simulink model "r_2x_8a_16x_4k_r352"  (Simulink 8.0) (continued)
== system r_2x_8a_16x_4k_r352 == (continued)
  Slice2 : xbsIndex_r4/Slice  nbits=1  boolean_output=on  mode=Lower Bit Location + Width  bit1=0  base1=MSB of Input  bit0=10  base0=LSB of Input  dbl_ovrd=off  block_type=slice
      settings: infoedit="Extracts a given range of bits from each input sample and presents it at the output.  The output type is ordinarily unsigned with binary point at zero, but can be Boolean when the slice is one bit wide.<P><P>Hardware notes: In hardware this block costs nothing."  block_version=10.1.3
  Slice3 : xbsIndex_r4/Slice  nbits=2  boolean_output=off  mode=Lower Bit Location + Width  bit1=0  base1=MSB of Input  bit0=1  base0=LSB of Input  dbl_ovrd=off  block_type=slice
      settings: infoedit="Extracts a given range of bits from each input sample and presents it at the output.  The output type is ordinarily unsigned with binary point at zero, but can be Boolean when the slice is one bit wide.<P><P>Hardware notes: In hardware this block costs nothing."  block_version=10.1.3
  Slice4 : xbsIndex_r4/Slice  nbits=1  boolean_output=on  mode=Upper Bit Location + Width  bit1=0  base1=MSB of Input  bit0=0  base0=LSB of Input  dbl_ovrd=off  block_type=slice
      settings: block_version=10.1.3
  Slice5 : xbsIndex_r4/Slice  nbits=6  boolean_output=off  mode=Lower Bit Location + Width  bit1=0  base1=MSB of Input  bit0=9  base0=LSB of Input  dbl_ovrd=off  block_type=slice
      settings: infoedit="Extracts a given range of bits from each input sample and presents it at the output.  The output type is ordinarily unsigned with binary point at zero, but can be Boolean when the slice is one bit wide.<P><P>Hardware notes: In hardware this block costs nothing."  block_version=10.1.3
  Slice6 : xbsIndex_r4/Slice  nbits=1  boolean_output=on  mode=Lower Bit Location + Width  bit1=0  base1=MSB of Input  bit0=12  base0=LSB of Input  dbl_ovrd=off  block_type=slice
      settings: block_version=10.1.3
  Slice7 : xbsIndex_r4/Slice  nbits=1  boolean_output=on  mode=Lower Bit Location + Width  bit1=0  base1=MSB of Input  bit0=8  base0=LSB of Input  dbl_ovrd=off  block_type=slice
      settings: infoedit="Extracts a given range of bits from each input sample and presents it at the output.  The output type is ordinarily unsigned with binary point at zero, but can be Boolean when the slice is one bit wide.<P><P>Hardware notes: In hardware this block costs nothing."  block_version=10.1.3
  Slice8 : xbsIndex_r4/Slice  nbits=1  boolean_output=on  mode=Lower Bit Location + Width  bit1=0  base1=MSB of Input  bit0=11  base0=LSB of Input  dbl_ovrd=off  block_type=slice
      settings: infoedit="Extracts a given range of bits from each input sample and presents it at the output.  The output type is ordinarily unsigned with binary point at zero, but can be Boolean when the slice is one bit wide.<P><P>Hardware notes: In hardware this block costs nothing."  block_version=10.1.3
  Slice9 : xbsIndex_r4/Slice  nbits=1  boolean_output=on  mode=Lower Bit Location + Width  bit1=0  base1=MSB of Input  bit0=15  base0=LSB of Input  dbl_ovrd=off  block_type=slice
      settings: infoedit="Extracts a given range of bits from each input sample and presents it at the output.  The output type is ordinarily unsigned with binary point at zero, but can be Boolean when the slice is one bit wide.<P><P>Hardware notes: In hardware this block costs nothing."  block_version=10.1.3
  Subsystem : Subsystem  mask{payload_len=32}
      mask prompts: payload_len "Payload length?"
      mask init:
      | packet_len = payload_len + 1;
      | packet_bits = ceil(log2(packet_len));
      | payload_bits = ceil(log2(payload_len));
  Subsystem1 : Subsystem
  Subsystem14 : Subsystem
  Subsystem2 : Subsystem
  Subsystem3 : Subsystem
  Subsystem4 : Subsystem
  Subsystem5 : Subsystem
  Subsystem6 : Subsystem
  Subsystem7 : Subsystem
  Terminator{1..27,30} (28 alike) : Terminator
  XSG core config : xps_library/XSG core config  Tag=xps:xsg  hw_sys=ROACH:sx95t  clk_src=arb_clk  ROACH_clk_src=arb_clk  ROACH2_clk_src=adc0_clk  clk_rate=210  sample_period=1  synthesis_tool=XST
  acc_len : xps_library/software register  Tag=xps:sw_reg  io_dir=From Processor  arith_type=Unsigned  bitwidth=32  bin_pt=0  sample_period=1  gw_name=r_2x_8a_16x_4k_r352_acc_len_user_data_out
  board_id : xps_library/software register  Tag=xps:sw_reg  io_dir=From Processor  arith_type=Unsigned  bitwidth=32  bin_pt=0  sample_period=1  gw_name=r_2x_8a_16x_4k_r352_board_id_user_data_out
  ctrl : xps_library/software register  Tag=xps:sw_reg  io_dir=From Processor  arith_type=Unsigned  bitwidth=32  bin_pt=0  sample_period=1  gw_name=r_2x_8a_16x_4k_r352_ctrl_user_data_out
  delay1 : xbsIndex_r4/Delay  rst=off  infoeditControl=Selection of Reset will increase slice count due to use of real FFs and instead of SRLs  en=off  latency=2  dbl_ovrd=off  reg_retiming=on  block_type=delay
  delay{2,4,6..17,19..20} (16 alike) : xbsIndex_r4/Delay  rst=off  infoeditControl=Selection of Reset will increase slice count due to use of real FFs and instead of SRLs  en=off  latency=1  dbl_ovrd=off  reg_retiming=on  block_type=delay
  delay5 : xbsIndex_r4/Delay  rst=off  infoeditControl=Selection of Reset will increase slice count due to use of real FFs and instead of SRLs  en=off  latency=2  dbl_ovrd=off  reg_retiming=on  block_type=delay
  dev_sel2 : xbsIndex_r4/Slice  nbits=1  boolean_output=off  mode=Lower Bit Location + Width  bit1=0  base1=MSB of Input  bit0=0  base0=LSB of Input  dbl_ovrd=off  block_type=slice
      settings: infoedit="Extracts a given range of bits from each input sample and presents it at the output.  The output type is ordinarily unsigned with binary point at zero, but can be Boolean when the slice is one bit wide.<P><P>Hardware notes: In hardware this block costs nothing."  block_version=10.1.3
  dev_sel3 : xbsIndex_r4/Slice  nbits=1  boolean_output=on  mode=Lower Bit Location + Width  bit1=0  base1=MSB of Input  bit0=0  base0=LSB of Input  dbl_ovrd=off  block_type=slice
      settings: infoedit="Extracts a given range of bits from each input sample and presents it at the output.  The output type is ordinarily unsigned with binary point at zero, but can be Boolean when the slice is one bit wide.<P><P>Hardware notes: In hardware this block costs nothing."  block_version=10.1.3
  dev_sel5 : xbsIndex_r4/Slice  nbits=2  boolean_output=off  mode=Lower Bit Location + Width  bit1=0  base1=MSB of Input  bit0=3  base0=LSB of Input  dbl_ovrd=off  block_type=slice
      settings: infoedit="Extracts a given range of bits from each input sample and presents it at the output.  The output type is ordinarily unsigned with binary point at zero, but can be Boolean when the slice is one bit wide.<P><P>Hardware notes: In hardware this block costs nothing."  block_version=10.1.3
  err_clear_sim{1..3,5..9} (8 alike) : Constant  Value=0
  front_panel : Subsystem  mask{in_step_period=16, desired_step_period=23}
      mask prompts: in_step_period "Input step period (2^?)"; desired_step_period "Desired step period (2^?)"
  gbe0 : xps_library/ten_Gbe_v2  Tag=xps:tengbe_v2  flavour=cx4  slot=1  port_r1=0  port_r2_cx4=0  port_r2_sfpp=0  rx_dist_ram=on  large_frames=off  show_param=on  pre_emph_r2=0.74  pre_emph=3  post_emph_r2=0.18  rxeqmix_r2=7  swing_r2=940  swing=800  fab_en=off  fab_mac=hex2dec('123456780000')  fab_ip=192*(2^24) + 168*(2^16) + 5*(2^8) + 20*(2^0)  fab_udp=10000  fab_gate=1  cpu_rx_en=on  cpu_tx_en=on
  gbe_err : Subsystem  mask{payload_len=32}
      mask prompts: payload_len "Packet payload length in 64-bit words?"
      mask init:
      | packet_len = payload_len + 1;
      | packet_bits = ceil(log2(packet_len));
      | payload_bits = ceil(log2(payload_len));
  gbe_out_ip : xps_library/software register  Tag=xps:sw_reg  io_dir=From Processor  arith_type=Unsigned  bitwidth=32  bin_pt=0  sample_period=1  gw_name=r_2x_8a_16x_4k_r352_gbe_out_ip_user_data_out
  gbe_out_port : xps_library/software register  Tag=xps:sw_reg  io_dir=From Processor  arith_type=Unsigned  bitwidth=32  bin_pt=0  sample_period=1  gw_name=r_2x_8a_16x_4k_r352_gbe_out_port_user_data_out
  gbe_rx_cnt0 : xps_library/software register  Tag=xps:sw_reg  io_dir=To Processor  arith_type=Unsigned  bitwidth=32  bin_pt=0  sample_period=1  gw_name=r_2x_8a_16x_4k_r352_gbe_rx_cnt0_user_data_in
  gbe_rx_err_cnt0 : xps_library/software register  Tag=xps:sw_reg  io_dir=To Processor  arith_type=Unsigned  bitwidth=32  bin_pt=0  sample_period=1  gw_name=r_2x_8a_16x_4k_r352_gbe_rx_err_cnt0_user_data_in
  gbe_tx_cnt0 : xps_library/software register  Tag=xps:sw_reg  io_dir=To Processor  arith_type=Unsigned  bitwidth=32  bin_pt=0  sample_period=1  gw_name=r_2x_8a_16x_4k_r352_gbe_tx_cnt0_user_data_in
  gbe_tx_err_cnt0 : xps_library/software register  Tag=xps:sw_reg  io_dir=To Processor  arith_type=Unsigned  bitwidth=32  bin_pt=0  sample_period=1  gw_name=r_2x_8a_16x_4k_r352_gbe_tx_err_cnt0_user_data_in
  last_missing_ant0 : xps_library/software register  Tag=xps:sw_reg  io_dir=To Processor  arith_type=Unsigned  bitwidth=32  bin_pt=0  sample_period=1  gw_name=r_2x_8a_16x_4k_r352_last_missing_ant0_user_data_in
  last_missing_ant1 : xps_library/software register  Tag=xps:sw_reg  io_dir=To Processor  arith_type=Unsigned  bitwidth=32  bin_pt=0  sample_period=1  gw_name=r_2x_8a_16x_4k_r352_last_missing_ant1_user_data_in
  led0_10gbe_fault : xps_library/gpio  Tag=xps:gpio  io_group=ROACH:led  io_dir=out  arith_type=Boolean  bitwidth=1  bin_pt=0  bit_index=0  sample_period=1  use_single_ended=off  use_ddr=off  reg_iob=off  reg_clk_phase=0  termination=None
      link override convert: arith_type=Boolean  n_bits=1  bin_pt=0
  led1_xaui_sync : xps_library/gpio  Tag=xps:gpio  io_group=ROACH:led  io_dir=out  arith_type=Boolean  bitwidth=1  bin_pt=0  bit_index=1  sample_period=1  use_single_ended=off  use_ddr=off  reg_iob=off  reg_clk_phase=0  termination=None
      link override convert: arith_type=Boolean  n_bits=1  bin_pt=0
  led2_x_sync : xps_library/gpio  Tag=xps:gpio  io_group=ROACH:led  io_dir=out  arith_type=Boolean  bitwidth=1  bin_pt=0  bit_index=2  sample_period=1  use_single_ended=off  use_ddr=off  reg_iob=off  reg_clk_phase=0  termination=None
      link override convert: arith_type=Boolean  n_bits=1  bin_pt=0
  led3_new_acc : xps_library/gpio  Tag=xps:gpio  io_group=ROACH:led  io_dir=out  arith_type=Boolean  bitwidth=1  bin_pt=0  bit_index=3  sample_period=1  use_single_ended=off  use_ddr=off  reg_iob=off  reg_clk_phase=0  termination=None
      link override convert: arith_type=Boolean  n_bits=1  bin_pt=0
  master_reset_sim4 : Constant  Value=2
  miss_cnt0 : Subsystem  mask{n_ants_bits=3, xeng_int_bits=7}
      mask prompts: n_ants_bits "Number of antennas in system (2^?)"; xeng_int_bits "X engine integration time (2^?)"
  miss_cnt1 : Subsystem  mask{n_ants_bits=3, xeng_int_bits=7}
      mask prompts: n_ants_bits "Number of antennas in system (2^?)"; xeng_int_bits "X engine integration time (2^?)"
  mux_out : Subsystem  mask{idle_clks=62000}
      mask prompts: idle_clks "Rate control. Idle clocks between packets"
      mask init:
      | idle_clks_bits = ceil(log2(idle_clks));
  negedge_delay{,1..4} (5 alike) : casper_library_misc/negedge_delay  pulse_len=2^23
  pack_out0 : Subsystem  mask(pkt_pack_mask){vector_len=147456/2, heap_len=147456/2*16*4, fifo_latency=2, spead_msw=64, spead_lsw=40, spead_item=6144, pkt_bits=9}
      mask prompts: vector_len "vector length"; heap_len "System heap size"; fifo_latency "Fifo Latency"; spead_msw "SPEAD flavour (MSw)"; spead_lsw "SPEAD flavour (LSw)"; spead_item "SPEAD item number"; pkt_bits "desired payload length (2^?) 64-bit words"
      mask help:
      | This block generates packets from a datastream.
      |
      | Packets are created with a fixed header followed by a user-specified number of 64-bit options.
      |
      | Requires a 64-bit data stream.
      |
      | See the documentation for further details.
      mask init:
      | vector_bits = ceil(log2(vector_len));
      | pkt_len=2^(pkt_bits);
  pack_out1 : Subsystem  mask(pkt_pack_mask){vector_len=147456/2, heap_len=147456/2*16*4, fifo_latency=2, spead_msw=64, spead_lsw=40, spead_item=6144, pkt_bits=9}
      mask prompts: vector_len "vector length"; heap_len "System heap size"; fifo_latency "Fifo Latency"; spead_msw "SPEAD flavour (MSw)"; spead_lsw "SPEAD flavour (LSw)"; spead_item "SPEAD item number"; pkt_bits "desired payload length (2^?) 64-bit words"
      mask help:
      | This block generates packets from a datastream.
      |
      | Packets are created with a fixed header followed by a user-specified number of 64-bit options.
      |
      | Requires a 64-bit data stream.
      |
      | See the documentation for further details.
      mask init:
      | vector_bits = ceil(log2(vector_len));
      | pkt_len=2^(pkt_bits);
  pack_out_cnt0 : xps_library/software register  Tag=xps:sw_reg  io_dir=To Processor  arith_type=Unsigned  bitwidth=32  bin_pt=0  sample_period=1  gw_name=r_2x_8a_16x_4k_r352_pack_out_cnt0_user_data_in
  pack_out_cnt1 : xps_library/software register  Tag=xps:sw_reg  io_dir=To Processor  arith_type=Unsigned  bitwidth=32  bin_pt=0  sample_period=1  gw_name=r_2x_8a_16x_4k_r352_pack_out_cnt1_user_data_in
  pack_out_err0 : xps_library/software register  Tag=xps:sw_reg  io_dir=To Processor  arith_type=Unsigned  bitwidth=32  bin_pt=0  sample_period=1  gw_name=r_2x_8a_16x_4k_r352_pack_out_err0_user_data_in
  pack_out_err1 : xps_library/software register  Tag=xps:sw_reg  io_dir=To Processor  arith_type=Unsigned  bitwidth=32  bin_pt=0  sample_period=1  gw_name=r_2x_8a_16x_4k_r352_pack_out_err1_user_data_in
  pkt_reord0 : Subsystem  mask{ant_bits=3, xeng_bits=4, chan_bits=12, x_int_bits=7, window_bits=3, min_sync_bits=10, share_bits=1, nx=0}
      mask prompts: ant_bits "Maximum Number of Antennas (2^?):"; xeng_bits "Number of Xengines in the system (2^?)"; chan_bits "Number of frequency channels in the system (2^?)"; x_int_bits "X Engine Integration Length (2^?):"; window_bits "Number of Windows to Buffer (2^?):"; min_sync_bits "Minimum sync period (2^n)"; share_bits "Number of Xengines on this link (2^?)"; nx "This is engine number"
      mask init:
      | sync_bits=max([min_sync_bits,chan_bits+x_int_bits+ant_bits-xeng_bits]);
      | %sync_bits=chan_bits+x_int_bits+ant_bits-xeng_bits;
      | fmtstr = sprintf('Xeng %d\nSync period: 2^%d\nPayload Len: %i', nx,sync_bits,2^(x_int_bits - 2));
      | set_param(gcb, 'AttributesFormatString', fmtstr);
      settings: AttributesFormatString=|
      | Xeng 0
      | Sync period: 2^18
      | Payload Len: 32
  pkt_reord1 : Subsystem  mask{ant_bits=3, xeng_bits=4, chan_bits=12, x_int_bits=7, window_bits=3, min_sync_bits=10, share_bits=1, nx=1}
      mask prompts: ant_bits "Maximum Number of Antennas (2^?):"; xeng_bits "Number of Xengines in the system (2^?)"; chan_bits "Number of frequency channels in the system (2^?)"; x_int_bits "X Engine Integration Length (2^?):"; window_bits "Number of Windows to Buffer (2^?):"; min_sync_bits "Minimum sync period (2^n)"; share_bits "Number of Xengines on this link (2^?)"; nx "This is engine number"
      mask init: same as r_2x_8a_16x_4k_r352/pkt_reord0
      settings: AttributesFormatString=|
      | Xeng 1
      | Sync period: 2^18
      | Payload Len: 32
  pkt_reord_cnt0 : xps_library/software register  Tag=xps:sw_reg  io_dir=To Processor  arith_type=Unsigned  bitwidth=32  bin_pt=0  sample_period=1  gw_name=r_2x_8a_16x_4k_r352_pkt_reord_cnt0_user_data_in
  pkt_reord_cnt1 : xps_library/software register  Tag=xps:sw_reg  io_dir=To Processor  arith_type=Unsigned  bitwidth=32  bin_pt=0  sample_period=1  gw_name=r_2x_8a_16x_4k_r352_pkt_reord_cnt1_user_data_in
  pkt_reord_err0 : xps_library/software register  Tag=xps:sw_reg  io_dir=To Processor  arith_type=Unsigned  bitwidth=32  bin_pt=0  sample_period=1  gw_name=r_2x_8a_16x_4k_r352_pkt_reord_err0_user_data_in
  pkt_reord_err1 : xps_library/software register  Tag=xps:sw_reg  io_dir=To Processor  arith_type=Unsigned  bitwidth=32  bin_pt=0  sample_period=1  gw_name=r_2x_8a_16x_4k_r352_pkt_reord_err1_user_data_in
  posedge2 : casper_library_misc/posedge  Tag=Rising Edge Detector
  prep_snap_rx0 : Subsystem
  rcs : Subsystem  AncestorBlock=casper_library_misc/rcs  mask(rcs){app_src=timestamp, lib_src=git, user_val=352}
      mask prompts: app_src "Application revision info to capture"; lib_src "CASPER libraries revision info to capture"; user_val "User specified value"
      mask help:
      | Embeds revision control information for the user design and libraries.
      | On failure to locate specified revision information, falls back to
      | timestamps (current time for libraries).
      | A user specified value is also stored.
      mask init:
      | rcs_init(gcb, ...
      |     'app_src', app_src, ...
      |     'lib_src', lib_src);
      mask TypeOptions app_src: timestamp | git | svn
      mask TypeOptions lib_src: timestamp | git | svn
  rx_cnt0 : xps_library/software register  Tag=xps:sw_reg  io_dir=To Processor  arith_type=Unsigned  bitwidth=32  bin_pt=0  sample_period=1  gw_name=r_2x_8a_16x_4k_r352_rx_cnt0_user_data_in
  rx_err_cnt0 : xps_library/software register  Tag=xps:sw_reg  io_dir=To Processor  arith_type=Unsigned  bitwidth=32  bin_pt=0  sample_period=1  gw_name=r_2x_8a_16x_4k_r352_rx_err_cnt0_user_data_in
  snap_descramble0 : Subsystem  AncestorBlock=casper_library_scopes/snapshot  mask(snapshot){storage=bram, dram_dimm=1, dram_clock=200, nsamples=10, data_width=32, offset=on, circap=on, value=off, use_dsp48=on}
      mask prompts: storage "Storage medium"; dram_dimm "DRAM dimm"; dram_clock "DRAM clock rate"; nsamples "Number of Samples (2^?)"; data_width "Data width"; offset "Start delay support"; circap "Circular capture support"; value "Extra value capture support"; use_dsp48 "Use DSP48s to implement counters"
      mask help:
      | Standard snap block, but with the following optional extras;
      |  1) Optionally delay the fabric trigger by a user-specified number of valids.
      |  2) Capture continuously after a trigger until a stop pulse is received. Data is captured into BRAM continuously, with old data being overwritten until a stop command is received. Then stop capturing when memory is filled. This enables you to get some data before and some data after the stop pulse. Use the 'tr_en_cnt' register to determine where you are in your vector. 'Addr' register's MSb now indicates 'busy capturing'.
      |  3) Optionally capture a value on an input port to a register at the same time as the first data sample.
      |  4) Implement counters using DSP48Es to save resources.
      mask init:
      | snapshot_init(gcb, ...
      |     'storage', storage, ...
      |     'dram_dimm', dram_dimm, ...
      |     'dram_clock', dram_clock, ...
      |     'nsamples', nsamples, ...
      |     'data_width', data_width, ...
      |     'offset', offset, ...
      |     'circap', circap, ...
      |     'value', value, ...
      |     'use_dsp48', use_dsp48);
      mask TypeOptions storage: bram | dram
      mask Callback storage: snapshot_callback();
      mask TypeOptions dram_dimm: 1 | 2 | 3 | 4
      mask TypeOptions dram_clock: 200 | 250 | 266 | 300 | 333
      mask TypeOptions data_width: 8 | 16 | 32 | 64 | 128
  snap_descramble1 : Subsystem  AncestorBlock=casper_library_scopes/snapshot  mask(snapshot){storage=bram, dram_dimm=1, dram_clock=200, nsamples=10, data_width=32, offset=on, circap=on, value=off, use_dsp48=on}
      mask prompts: storage "Storage medium"; dram_dimm "DRAM dimm"; dram_clock "DRAM clock rate"; nsamples "Number of Samples (2^?)"; data_width "Data width"; offset "Start delay support"; circap "Circular capture support"; value "Extra value capture support"; use_dsp48 "Use DSP48s to implement counters"
      mask help:
      | Standard snap block, but with the following optional extras;
      |  1) Optionally delay the fabric trigger by a user-specified number of valids.
      |  2) Capture continuously after a trigger until a stop pulse is received. Data is captured into BRAM continuously, with old data being overwritten until a stop command is received. Then stop capturing when memory is filled. This enables you to get some data before and some data after the stop pulse. Use the 'tr_en_cnt' register to determine where you are in your vector. 'Addr' register's MSb now indicates 'busy capturing'.
      |  3) Optionally capture a value on an input port to a register at the same time as the first data sample.
      |  4) Implement counters using DSP48Es to save resources.
      mask init: same as r_2x_8a_16x_4k_r352/snap_descramble0
      mask TypeOptions storage: bram | dram
      mask Callback storage: snapshot_callback();
      mask TypeOptions dram_dimm: 1 | 2 | 3 | 4
      mask TypeOptions dram_clock: 200 | 250 | 266 | 300 | 333
      mask TypeOptions data_width: 8 | 16 | 32 | 64 | 128
  snap_gbe_rx0 : Subsystem  AncestorBlock=casper_library_scopes/snapshot  mask(snapshot){storage=bram, dram_dimm=1, dram_clock=200, nsamples=10, data_width=128, offset=off, circap=off, value=off, use_dsp48=on}
      mask prompts: storage "Storage medium"; dram_dimm "DRAM dimm"; dram_clock "DRAM clock rate"; nsamples "Number of Samples (2^?)"; data_width "Data width"; offset "Start delay support"; circap "Circular capture support"; value "Extra value capture support"; use_dsp48 "Use DSP48s to implement counters"
      mask help:
      | Standard snap block, but with the following optional extras;
      |  1) Optionally delay the fabric trigger by a user-specified number of valids.
      |  2) Capture continuously after a trigger until a stop pulse is received. Data is captured into BRAM continuously, with old data being overwritten until a stop command is received. Then stop capturing when memory is filled. This enables you to get some data before and some data after the stop pulse. Use the 'tr_en_cnt' register to determine where you are in your vector. 'Addr' register's MSb now indicates 'busy capturing'.
      |  3) Optionally capture a value on an input port to a register at the same time as the first data sample.
      |  4) Implement counters using DSP48Es to save resources.
      mask init: same as r_2x_8a_16x_4k_r352/snap_descramble0
      mask TypeOptions storage: bram | dram
      mask Callback storage: snapshot_callback();
      mask TypeOptions dram_dimm: 1 | 2 | 3 | 4
      mask TypeOptions dram_clock: 200 | 250 | 266 | 300 | 333
      mask TypeOptions data_width: 8 | 16 | 32 | 64 | 128
  snap_gbe_tx0 : Subsystem  AncestorBlock=casper_library_scopes/snapshot  mask(snapshot){storage=bram, dram_dimm=1, dram_clock=200, nsamples=10, data_width=128, offset=off, circap=off, value=off, use_dsp48=on}
      mask prompts: storage "Storage medium"; dram_dimm "DRAM dimm"; dram_clock "DRAM clock rate"; nsamples "Number of Samples (2^?)"; data_width "Data width"; offset "Start delay support"; circap "Circular capture support"; value "Extra value capture support"; use_dsp48 "Use DSP48s to implement counters"
      mask help:
      | Standard snap block, but with the following optional extras;
      |  1) Optionally delay the fabric trigger by a user-specified number of valids.
      |  2) Capture continuously after a trigger until a stop pulse is received. Data is captured into BRAM continuously, with old data being overwritten until a stop command is received. Then stop capturing when memory is filled. This enables you to get some data before and some data after the stop pulse. Use the 'tr_en_cnt' register to determine where you are in your vector. 'Addr' register's MSb now indicates 'busy capturing'.
      |  3) Optionally capture a value on an input port to a register at the same time as the first data sample.
      |  4) Implement counters using DSP48Es to save resources.
      mask init: same as r_2x_8a_16x_4k_r352/snap_descramble0
      mask TypeOptions storage: bram | dram
      mask Callback storage: snapshot_callback();
      mask TypeOptions dram_dimm: 1 | 2 | 3 | 4
      mask TypeOptions dram_clock: 200 | 250 | 266 | 300 | 333
      mask TypeOptions data_width: 8 | 16 | 32 | 64 | 128
  snap_rx0 : Subsystem  AncestorBlock=casper_library_scopes/snapshot  mask(snapshot){storage=bram, dram_dimm=1, dram_clock=200, nsamples=10, data_width=128, offset=off, circap=off, value=off, use_dsp48=on}
      mask prompts: storage "Storage medium"; dram_dimm "DRAM dimm"; dram_clock "DRAM clock rate"; nsamples "Number of Samples (2^?)"; data_width "Data width"; offset "Start delay support"; circap "Circular capture support"; value "Extra value capture support"; use_dsp48 "Use DSP48s to implement counters"
      mask help:
      | Standard snap block, but with the following optional extras;
      |  1) Optionally delay the fabric trigger by a user-specified number of valids.
      |  2) Capture continuously after a trigger until a stop pulse is received. Data is captured into BRAM continuously, with old data being overwritten until a stop command is received. Then stop capturing when memory is filled. This enables you to get some data before and some data after the stop pulse. Use the 'tr_en_cnt' register to determine where you are in your vector. 'Addr' register's MSb now indicates 'busy capturing'.
      |  3) Optionally capture a value on an input port to a register at the same time as the first data sample.
      |  4) Implement counters using DSP48Es to save resources.
      mask init: same as r_2x_8a_16x_4k_r352/snap_descramble0
      mask TypeOptions storage: bram | dram
      mask Callback storage: snapshot_callback();
      mask TypeOptions dram_dimm: 1 | 2 | 3 | 4
      mask TypeOptions dram_clock: 200 | 250 | 266 | 300 | 333
      mask TypeOptions data_width: 8 | 16 | 32 | 64 | 128
  snap_vacc0 : Subsystem  AncestorBlock=casper_library_scopes/snapshot  mask(snapshot){storage=bram, dram_dimm=1, dram_clock=200, nsamples=10, data_width=32, offset=on, circap=on, value=off, use_dsp48=on}
      mask prompts: storage "Storage medium"; dram_dimm "DRAM dimm"; dram_clock "DRAM clock rate"; nsamples "Number of Samples (2^?)"; data_width "Data width"; offset "Start delay support"; circap "Circular capture support"; value "Extra value capture support"; use_dsp48 "Use DSP48s to implement counters"
      mask help:
      | Standard snap block, but with the following optional extras;
      |  1) Optionally delay the fabric trigger by a user-specified number of valids.
      |  2) Capture continuously after a trigger until a stop pulse is received. Data is captured into BRAM continuously, with old data being overwritten until a stop command is received. Then stop capturing when memory is filled. This enables you to get some data before and some data after the stop pulse. Use the 'tr_en_cnt' register to determine where you are in your vector. 'Addr' register's MSb now indicates 'busy capturing'.
      |  3) Optionally capture a value on an input port to a register at the same time as the first data sample.
      |  4) Implement counters using DSP48Es to save resources.
      mask init: same as r_2x_8a_16x_4k_r352/snap_descramble0
      mask TypeOptions storage: bram | dram
      mask Callback storage: snapshot_callback();
      mask TypeOptions dram_dimm: 1 | 2 | 3 | 4
      mask TypeOptions dram_clock: 200 | 250 | 266 | 300 | 333
      mask TypeOptions data_width: 8 | 16 | 32 | 64 | 128
  snap_xeng0 : Subsystem  AncestorBlock=casper_library_scopes/snapshot  mask(snapshot){storage=bram, dram_dimm=1, dram_clock=200, nsamples=10, data_width=32, offset=on, circap=off, value=off, use_dsp48=on}
      mask prompts: storage "Storage medium"; dram_dimm "DRAM dimm"; dram_clock "DRAM clock rate"; nsamples "Number of Samples (2^?)"; data_width "Data width"; offset "Start delay support"; circap "Circular capture support"; value "Extra value capture support"; use_dsp48 "Use DSP48s to implement counters"
      mask help:
      | Standard snap block, but with the following optional extras;
      |  1) Optionally delay the fabric trigger by a user-specified number of valids.
      |  2) Capture continuously after a trigger until a stop pulse is received. Data is captured into BRAM continuously, with old data being overwritten until a stop command is received. Then stop capturing when memory is filled. This enables you to get some data before and some data after the stop pulse. Use the 'tr_en_cnt' register to determine where you are in your vector. 'Addr' register's MSb now indicates 'busy capturing'.
      |  3) Optionally capture a value on an input port to a register at the same time as the first data sample.
      |  4) Implement counters using DSP48Es to save resources.
      mask init: same as r_2x_8a_16x_4k_r352/snap_descramble0
      mask TypeOptions storage: bram | dram
      mask Callback storage: snapshot_callback();
      mask TypeOptions dram_dimm: 1 | 2 | 3 | 4
      mask TypeOptions dram_clock: 200 | 250 | 266 | 300 | 333
      mask TypeOptions data_width: 8 | 16 | 32 | 64 | 128
  tvg_sel : xps_library/software register  Tag=xps:sw_reg  io_dir=From Processor  arith_type=Unsigned  bitwidth=32  bin_pt=0  sample_period=1  gw_name=r_2x_8a_16x_4k_r352_tvg_sel_user_data_out
  unpack : Subsystem  mask{payload_len=32}
      mask prompts: payload_len "Expected payload length (in 64bit words)"
      mask init:
      | payload_bits = ceil(log2(payload_len)+1);
      | fmtstr = sprintf('Payload Len: %i', payload_len);
      | set_param(gcb, 'AttributesFormatString', fmtstr);
      settings: AttributesFormatString=Payload Len: 32
  vacc0 : Subsystem  mask{ants_bits=3, freq_bits=12, xeng_bits=4, which_qdr=qdr0}
      mask prompts: ants_bits "Number of antennas (2^n)"; freq_bits "Number of frequency channels (2^n)"; xeng_bits "Number of X engines (2^n)"; which_qdr "QDR"
      mask help: Hardcoded for X engines with a demux of 8!
      mask init:
      | vector_len = (2^ants_bits)*(2^ants_bits +1) /2 *8 * 2^(freq_bits-xeng_bits);
      | set_param([gcb, '/qv'], 'which_qdr', which_qdr);
      | fmtstr = sprintf('Vector Length: %d', vector_len);
      | set_param(gcb, 'AttributesFormatString', fmtstr);
      settings: AttributesFormatString=Vector Length: 73728
      mask TypeOptions which_qdr: qdr0 | qdr1
  vacc1 : Subsystem  mask{ants_bits=3, freq_bits=12, xeng_bits=4, which_qdr=qdr1}
      mask prompts: ants_bits "Number of antennas (2^n)"; freq_bits "Number of frequency channels (2^n)"; xeng_bits "Number of X engines (2^n)"; which_qdr "QDR"
      mask help: Hardcoded for X engines with a demux of 8!
      mask init: same as r_2x_8a_16x_4k_r352/vacc0
      settings: AttributesFormatString=Vector Length: 73728
      mask TypeOptions which_qdr: qdr0 | qdr1
  vacc_cnt0 : xps_library/software register  Tag=xps:sw_reg  io_dir=To Processor  arith_type=Unsigned  bitwidth=32  bin_pt=0  sample_period=1  gw_name=r_2x_8a_16x_4k_r352_vacc_cnt0_user_data_in
  vacc_cnt1 : xps_library/software register  Tag=xps:sw_reg  io_dir=To Processor  arith_type=Unsigned  bitwidth=32  bin_pt=0  sample_period=1  gw_name=r_2x_8a_16x_4k_r352_vacc_cnt1_user_data_in
  vacc_err_cnt0 : xps_library/software register  Tag=xps:sw_reg  io_dir=To Processor  arith_type=Unsigned  bitwidth=32  bin_pt=0  sample_period=1  gw_name=r_2x_8a_16x_4k_r352_vacc_err_cnt0_user_data_in
  vacc_err_cnt1 : xps_library/software register  Tag=xps:sw_reg  io_dir=To Processor  arith_type=Unsigned  bitwidth=32  bin_pt=0  sample_period=1  gw_name=r_2x_8a_16x_4k_r352_vacc_err_cnt1_user_data_in
  vacc_ld_status0 : xps_library/software register  Tag=xps:sw_reg  io_dir=To Processor  arith_type=Unsigned  bitwidth=32  bin_pt=0  sample_period=1  gw_name=r_2x_8a_16x_4k_r352_vacc_ld_status0_user_data_in
  vacc_ld_status1 : xps_library/software register  Tag=xps:sw_reg  io_dir=To Processor  arith_type=Unsigned  bitwidth=32  bin_pt=0  sample_period=1  gw_name=r_2x_8a_16x_4k_r352_vacc_ld_status1_user_data_in
  vacc_monitor : Subsystem
  vacc_monitor1 : Subsystem
  vacc_monitor2 : Subsystem
  vacc_monitor3 : Subsystem
  vacc_time_lsw : xps_library/software register  Tag=xps:sw_reg  io_dir=From Processor  arith_type=Unsigned  bitwidth=32  bin_pt=0  sample_period=1  gw_name=r_2x_8a_16x_4k_r352_vacc_time_lsw_user_data_out
  vacc_time_msw : xps_library/software register  Tag=xps:sw_reg  io_dir=From Processor  arith_type=Unsigned  bitwidth=32  bin_pt=0  sample_period=1  gw_name=r_2x_8a_16x_4k_r352_vacc_time_msw_user_data_out
  vacc_tvg0 : Subsystem  mask{len=147456/2}
      mask prompts: len "Number of Vectors"
      mask init:
      | fmtstr = sprintf('Vector Length: %d', len);
      | set_param(gcb, 'AttributesFormatString', fmtstr);
      settings: AttributesFormatString=Vector Length: 73728
  vacc_tvg1 : Subsystem  mask{len=147456/2}
      mask prompts: len "Number of Vectors"
      mask init:
      | fmtstr = sprintf('Vector Length: %d', len);
      | set_param(gcb, 'AttributesFormatString', fmtstr);
      settings: AttributesFormatString=Vector Length: 73728
  xeng0 : Subsystem  mask(xeng){n_ants=8, n_bits=4, acc_len=128, demux_factor=8, add_latency=1, mult_latency=2, bram_latency=2, use_ded_mult=1, use_bram_delay=1}
      mask prompts: n_ants "Number of antennas"; n_bits "Bit-width of samples in"; acc_len "Accumulation length"; add_latency "Adder latency"; mult_latency "Multiplier latency"; bram_latency "BRAM latency"; use_ded_mult "Implementation: Multiplier type (0=slices 1=embedded 2=bram)"; use_bram_delay "Implementation: Delay type (0=SLR, 1=BRAM)"
      mask help: CASPER X engine with added internal valid data masking functionality, descramble and integral demux. Based on Aaron Parsons' and Lynn Urry's original X engine design.
      mask init:
      | xeng_init(gcb, ...
      |     'n_ants', n_ants, ...
      |     'n_bits', n_bits, ...
      |     'acc_len', acc_len, ...
      |     'demux_factor', demux_factor, ...
      |     'add_latency', add_latency, ...
      |     'mult_latency', mult_latency, ...
      |     'bram_latency', bram_latency, ...
      |     'use_ded_mult', use_ded_mult, ...
      |     'use_bram_delay', use_bram_delay);
      settings: AttributesFormatString=n_ant=8, bits=4, mult=1, bram=1
      mask TypeOptions demux_factor: 1 | 2 | 4 | 8
  xeng1 : Subsystem  mask(xeng){n_ants=8, n_bits=4, acc_len=128, demux_factor=8, add_latency=1, mult_latency=2, bram_latency=2, use_ded_mult=1, use_bram_delay=1}
      mask prompts: n_ants "Number of antennas"; n_bits "Bit-width of samples in"; acc_len "Accumulation length"; add_latency "Adder latency"; mult_latency "Multiplier latency"; bram_latency "BRAM latency"; use_ded_mult "Implementation: Multiplier type (0=slices 1=embedded 2=bram)"; use_bram_delay "Implementation: Delay type (0=SLR, 1=BRAM)"
      mask help: CASPER X engine with added internal valid data masking functionality, descramble and integral demux. Based on Aaron Parsons' and Lynn Urry's original X engine design.
      mask init: same as r_2x_8a_16x_4k_r352/xeng0
      settings: AttributesFormatString=n_ant=8, bits=4, mult=1, bram=1
      mask TypeOptions demux_factor: 1 | 2 | 4 | 8
  xeng_tvg0 : Subsystem  AncestorBlock=casper_library/Correlator/xeng_tvg  mask{ant_bits=3, x_int_bits=7, sync_period=19}
      mask prompts: ant_bits "Number of Antennas (2^?):"; x_int_bits "X Integration Length (2^?):"; sync_period "Sync Pulse Period (2^?):"
  xeng_tvg1 : Subsystem  AncestorBlock=casper_library/Correlator/xeng_tvg  mask{ant_bits=3, x_int_bits=7, sync_period=19}
      mask prompts: ant_bits "Number of Antennas (2^?):"; x_int_bits "X Integration Length (2^?):"; sync_period "Sync Pulse Period (2^?):"
  xstatus0 : xps_library/software register  Tag=xps:sw_reg  io_dir=To Processor  arith_type=Unsigned  bitwidth=32  bin_pt=0  sample_period=1  gw_name=r_2x_8a_16x_4k_r352_xstatus0_user_data_in
  xstatus1 : xps_library/software register  Tag=xps:sw_reg  io_dir=To Processor  arith_type=Unsigned  bitwidth=32  bin_pt=0  sample_period=1  gw_name=r_2x_8a_16x_4k_r352_xstatus1_user_data_in
  wires:
  gbe_err/1(tx_cnt) -> Delay63/1(d)
  Subsystem/1(rx_cnt) -> Delay65/1(d)
  [cnt_rst] -> gbe_err/1(rst)
  [cnt_rst] -> Subsystem/1(rst)
  Subsystem5/1(rx_cnt) -> Delay66/1(d)
  [cnt_rst] -> Subsystem5/1(rst)
  unpack/2(valid) -> Delay37/1(d), Delay141/1(d), Delay80/1(d)
  unpack/4(mcnt) -> Delay39/1(d), Delay143/1(d)
  unpack/1(dout) -> Delay36/1(d), Delay140/1(d)
  unpack/3(eof) -> Delay38/1(d), Delay142/1(d), Delay81/1(d)
  unpack/5(ant) -> Delay35/1(d), Delay144/1(d)
  miss_cnt0/2(err_cnt) -> Delay62/1(d)
  [cnt_rst] -> miss_cnt0/1(rst)
  unpack/8(ip) -> Delay146/1(d)
  Delay36/1(q) -> pkt_reord0/1(din), pkt_reord1/1(din)
  Delay37/1(q) -> pkt_reord0/2(valid_in), pkt_reord1/2(valid_in)
  Delay38/1(q) -> pkt_reord0/3(eof), pkt_reord1/3(eof)
  Delay39/1(q) -> pkt_reord0/4(mcnt), pkt_reord1/4(mcnt)
  Delay35/1(q) -> pkt_reord0/5(ant), pkt_reord1/5(ant)
  Subsystem14/1(data) -> Delay41/1(d)
  Delay41/1(q) -> snap_descramble0/1(din)
  Subsystem14/2(we) -> Delay42/1(d)
  Subsystem14/3(trig) -> Delay43/1(d)
  Delay40/1(q) -> pkt_reord0/6(flag), pkt_reord1/6(flag)
  gbe_err/2(err_cnt) -> Delay64/1(d)
  Subsystem5/2(err_cnt) -> Delay67/1(d)
  miss_cnt0/1(spectr_cnt) -> Delay61/1(d)
  gbe_tx_cnt0/1 -> Terminator13/1
  gbe_tx_err_cnt0/1 -> Terminator14/1
  gbe_rx_cnt0/1 -> Terminator15/1
  rx_cnt0/1 -> Terminator16/1
  rx_err_cnt0/1 -> Terminator17/1
  pkt_reord_cnt0/1 -> Terminator25/1
  pkt_reord_err0/1 -> Terminator26/1
  Delay61/1(q) -> pkt_reord_cnt0/1
  Delay62/1(q) -> pkt_reord_err0/1
  Delay66/1(q) -> rx_cnt0/1
  Delay67/1(q) -> rx_err_cnt0/1
  Delay63/1(q) -> gbe_tx_cnt0/1
  Delay64/1(q) -> gbe_tx_err_cnt0/1
  Delay65/1(q) -> gbe_rx_cnt0/1
  pkt_reord0/4(mcnt_out) -> Delay26/1(d), Subsystem14/4(mcnt)
  pkt_reord0/1(sync_out) -> Delay20/1(d), [x_sync0], miss_cnt0/2(sync), Subsystem14/1(sync)
  pkt_reord0/3(valid_out) -> miss_cnt0/3(valid), Delay22/1(d), Subsystem14/3(valid)
  pkt_reord0/5(rcvd) -> miss_cnt0/4(rcvd), Subsystem14/6(rcvd)
  err_clear_sim3/1 -> board_id/1
  board_id/1 -> Delay72/1(d)
  Delay72/1(q) -> [board_id]
  master_reset_sim4/1 -> acc_len/1
  acc_len/1 -> [acc_len]
  tvg_sel/1 -> Delay89/1(d)
  Delay89/1(q) -> dev_sel2/1, Slice3/1, dev_sel5/1, Slice5/1
  dev_sel2/1 -> [xaui_tvg_sel]
  Slice3/1 -> [descr_tvg_sel]
  dev_sel5/1 -> [xeng_tvg_sel]
  Slice5/1 -> [vacc_tvg_sel]
  err_clear_sim2/1 -> Subsystem2/5(data_sel)
  err_clear_sim6/1 -> Subsystem2/4(valid_sel)
  err_clear_sim7/1 -> Subsystem2/3(inj_vector)
  err_clear_sim8/1 -> Subsystem2/2(inj_counter)
  Pulse Generator1/1 -> Subsystem2/1(new_acc)
  err_clear_sim9/1 -> Subsystem2/6(run)
  Subsystem2/1(Out1) -> tvg_sel/1
  Pulse Generator2/1 -> ctrl/1
  ctrl/1 -> Delay19/1(d)
  dev_sel3/1 -> Delay88/1(d)
  Slice9/1 -> Delay78/1(d)
  Slice7/1 -> posedge2/1
  posedge2/1 -> Delay58/1(d)
  Delay19/1(q) -> dev_sel3/1, Slice7/1, Slice1/1, Slice2/1, Slice8/1, Slice9/1, Slice14/1, Slice6/1, Slice10/1
  Delay58/1(q) -> [cnt_rst]
  Delay78/1(q) -> [gbe_rst]
  Delay88/1(q) -> [vacc_rst]
  Slice1/1 -> Delay60/1(d)
  Delay60/1(q) -> [gbe_enable]
  Delay17/1(q) -> negedge_delay/1
  [x_sync0] -> Delay17/1(d)
  led2_x_sync/1 -> Terminator30/1
  Constant3/1 -> Inverter2/1
  led1_xaui_sync/1 -> Terminator18/1
  led0_10gbe_fault/1 -> Terminator19/1
  Delay140/1(q) -> prep_snap_rx0/1(data_in)
  Delay141/1(q) -> prep_snap_rx0/2(valid_in)
  Delay142/1(q) -> prep_snap_rx0/3(eof_in)
  Delay143/1(q) -> prep_snap_rx0/4(mcnt)
  Delay144/1(q) -> prep_snap_rx0/5(ant)
  Delay145/1(q) -> prep_snap_rx0/6(flag)
  Delay146/1(q) -> prep_snap_rx0/7(ip_in)
  Delay147/1(q) -> prep_snap_rx0/8(loop_ack)
  Delay148/1(q) -> prep_snap_rx0/9(gbe_ack)
  Delay8/1(q) -> Subsystem4/7(bad_frame)
  Delay91/1(q) -> snap_descramble0/4(stop)
  Subsystem14/4(stop) -> Delay91/1(d)
  xeng_tvg0/1(sync_out) -> Delay56/1(d)
  xeng_tvg0/2(data_out) -> Delay73/1(d)
  xeng_tvg0/3(valid_out) -> Delay74/1(d)
  xeng0/1(sync_out) -> vacc_tvg0/2(sync_in), snap_xeng0/3(trig)
  xeng0/2(acc) -> 16b_32b/1(In1)
  xeng0/3(valid) -> snap_xeng0/2(we), vacc_tvg0/4(valid_in)
  [xeng_tvg_sel] -> xeng_tvg0/1(tvg_sel)
  [vacc_tvg_sel] -> vacc_tvg0/1(tvg_sel)
  Delay151/1(q) -> Delay83/1(d)
  Delay20/1(q) -> xeng_tvg0/2(sync)
  Delay21/1(q) -> xeng_tvg0/3(data_in)
  Delay22/1(q) -> xeng_tvg0/4(valid_in)
  Delay26/1(q) -> Delay151/1(d)
  [loopback_mux_rst] -> unpack/1(rst)
  gbe_rx_err_cnt0/1 -> Terminator7/1
  Delay2/1(q) -> gbe_rx_err_cnt0/1
  Subsystem/2(rx_err_cnt) -> Delay2/1(d)
  Slice2/1 -> Delay117/1(d)
  Delay117/1(q) -> [loopback_mux_rst]
  Delay118/1(q) -> negedge_delay1/1
  led3_new_acc/1 -> Terminator9/1
  Slice8/1 -> Delay122/1(d)
  Delay122/1(q) -> [gbe_out_rst]
  16b_32b/1(Out1) -> snap_xeng0/1(din), vacc_tvg0/3(data_in)
  miss_cnt0/3(last_missed_ant) -> Delay130/1(d)
  Slice14/1 -> Delay100/1(d)
  Delay100/1(q) -> [gbe_out_enable]
  unpack/6(flag) -> Delay40/1(d), Delay145/1(d)
  unpack/7(err) -> Delay82/1(d), [rx_bad_pkt]
  Delay12/1(q) -> Subsystem4/8(overrun)
  [gbe_up] -> Delay87/1(d)
  [gbe_rx] -> Delay86/1(d)
  [gbe_rxip] -> Delay75/1(d)
  [gbe_rxvld] -> Delay76/1(d)
  [gbe_rxdata] -> Delay79/1(d)
  [gbe_rxeof] -> Delay24/1(d)
  Delay87/1(q) -> Subsystem4/1(led_up)
  Delay86/1(q) -> Subsystem4/2(led_rx)
  Delay79/1(q) -> unpack/2(gbe_din)
  Delay76/1(q) -> unpack/3(gbe_valid)
  Delay75/1(q) -> unpack/4(gbe_ip)
  Delay24/1(q) -> unpack/5(gbe_eof)
  vacc_tvg0/1(sync_out) -> vacc0/5(sync_in)
  vacc_tvg0/2(data_out) -> vacc0/6(data_in)
  vacc_tvg0/3(valid_out) -> vacc0/7(we)
  [acc_len] -> vacc0/4(acc_len)
  xeng0/4(mcnt_out) -> delay5/1
  vacc_cnt0/1 -> Terminator10/1
  vacc_monitor/2(spec_cnt) -> vacc_cnt0/1
  vacc_err_cnt0/1 -> Terminator1/1
  vacc_monitor/1(error_cnt) -> vacc_err_cnt0/1
  vacc0/1(new_acc) -> [new_acc0]
  [cnt_rst] -> vacc_monitor/1(cnt_rst)
  vacc0/7(timestamp) -> delay7/1
  [cnt_rst] -> miss_cnt1/1(rst)
  xeng_tvg1/1(sync_out) -> Delay85/1(d)
  xeng_tvg1/2(data_out) -> Delay92/1(d)
  xeng_tvg1/3(valid_out) -> Delay94/1(d)
  xeng1/1(sync_out) -> vacc_tvg1/2(sync_in)
  xeng1/2(acc) -> 16b_32b1/1(In1)
  xeng1/3(valid) -> vacc_tvg1/4(valid_in)
  [xeng_tvg_sel] -> xeng_tvg1/1(tvg_sel)
  [vacc_tvg_sel] -> vacc_tvg1/1(tvg_sel)
  Delay30/1(q) -> Delay95/1(d)
  Delay49/1(q) -> xeng_tvg1/2(sync)
  Delay50/1(q) -> xeng_tvg1/3(data_in)
  Delay51/1(q) -> xeng_tvg1/4(valid_in)
  Delay52/1(q) -> Delay30/1(d)
  16b_32b1/1(Out1) -> vacc_tvg1/3(data_in)
  vacc_cnt1/1 -> Terminator20/1
  vacc_monitor1/2(spec_cnt) -> vacc_cnt1/1
  vacc_err_cnt1/1 -> Terminator8/1
  vacc_monitor1/1(error_cnt) -> vacc_err_cnt1/1
  [gbe_up] -> Subsystem3/5(link_up)
  [gbe_tx] -> Subsystem3/6(led_tx)
  [gbe_txd] -> Subsystem3/1(data_in)
  [gbe_txvld] -> Subsystem3/2(valid_in)
  [gbe_txip] -> Subsystem3/3(ip_in)
  [gbe_txeof] -> Subsystem3/4(eof_in)
  [gbe_rxvld] -> Delay25/1(d)
  [gbe_rxeof] -> Delay18/1(d)
  Delay25/1(q) -> Subsystem/2(valid)
  Delay18/1(q) -> Subsystem/3(eof)
  [gbe_rx_bad] -> Delay6/1(d)
  [gbe_rx_over] -> Delay7/1(d)
  [gbe_rxip] -> Delay53/1(d)
  [gbe_rxvld] -> Delay59/1(d)
  [gbe_rxdata] -> Delay70/1(d)
  [gbe_rxeof] -> Delay29/1(d)
  Delay70/1(q) -> Subsystem4/3(data_in)
  Delay59/1(q) -> Subsystem4/4(valid_in)
  Delay53/1(q) -> Subsystem4/5(ip_in)
  Delay29/1(q) -> Subsystem4/6(eof_in)
  [gbe_rxack] -> Subsystem4/9(ack_in)
  Delay80/1(q) -> Subsystem5/2(we)
  Delay81/1(q) -> Subsystem5/3(eof)
  Delay82/1(q) -> Subsystem5/4(flag)
  [gbe_tx_over] -> gbe_err/4(overflow)
  [gbe_txeof] -> gbe_err/3(eof)
  [gbe_txvld] -> gbe_err/2(valid)
  Delay6/1(q) -> Subsystem/4(rx_bad_frame)
  Delay7/1(q) -> Subsystem/5(rx_overflow)
  [gbe_rx_bad] -> Delay8/1(d)
  [gbe_rx_over] -> Delay12/1(d)
  [gbe_tx_full] -> Subsystem3/7(tx_full)
  [gbe_tx_over] -> Subsystem3/8(tx_over)
  Delay56/1(q) -> xeng0/1(sync_in)
  Delay73/1(q) -> xeng0/2(ant)
  Delay74/1(q) -> xeng0/3(window_valid)
  Delay83/1(q) -> xeng0/4(mcnt_in)
  Delay85/1(q) -> xeng1/1(sync_in)
  Delay92/1(q) -> xeng1/2(ant)
  Delay94/1(q) -> xeng1/3(window_valid)
  Delay95/1(q) -> xeng1/4(mcnt_in)
  [gbe_out_rst] -> delay8/1
  [gbe_txd] -> delay9/1
  [gbe_txvld] -> delay10/1
  [gbe_txip] -> delay11/1
  [gbe_out_txport] -> delay12/1
  [gbe_txeof] -> delay13/1
  [gbe_rxack] -> delay14/1
  [gbe_rxack] -> delay15/1
  gbe0/1 -> [gbe_up]
  gbe0/2 -> [gbe_rx]
  gbe0/3 -> [gbe_tx]
  gbe0/4 -> [gbe_tx_full]
  gbe0/5 -> [gbe_tx_over]
  gbe0/12 -> [gbe_rx_over]
  gbe0/11 -> [gbe_rx_bad]
  gbe0/6 -> [gbe_rxdata]
  gbe0/7 -> [gbe_rxvld]
  gbe0/8 -> [gbe_rxip]
  gbe0/9 -> [gbe_rxport]
  gbe0/10 -> [gbe_rxeof]
  Constant12/1 -> vacc_time_msw/1
  Constant6/1 -> vacc_time_lsw/1
  vacc_time_msw/1 -> Slice4/1, Slice13/1
  Slice13/1 -> Concat12/1
  vacc_time_lsw/1 -> Concat12/2
  Slice4/1 -> [vacc_ld_arm]
  Concat12/1 -> [vacc_ld_time]
  [vacc_ld_arm] -> vacc0/2(arm)
  [vacc_ld_time] -> vacc0/3(load_time)
  [vacc_rst] -> vacc0/1(rst)
  vacc_ld_status0/1 -> Terminator4/1
  vacc_tvg1/1(sync_out) -> vacc1/5(sync_in)
  vacc_tvg1/2(data_out) -> vacc1/6(data_in)
  vacc_tvg1/3(valid_out) -> vacc1/7(we)
  [acc_len] -> vacc1/4(acc_len)
  xeng1/4(mcnt_out) -> delay1/1
  [vacc_ld_arm] -> vacc1/2(arm)
  [vacc_ld_time] -> vacc1/3(load_time)
  [vacc_rst] -> vacc1/1(rst)
  vacc_ld_status1/1 -> Terminator3/1
  delay5/1 -> vacc0/8(pcnt_in)
  delay1/1 -> vacc1/8(pcnt_in)
  vacc0/10(error_out) -> [vacc_err0]
  [new_acc0] -> vacc_monitor/2(new_acc)
  [vacc_err0] -> vacc_monitor/3(error)
  pkt_reord0/2(data_out) -> Delay21/1(d), Subsystem14/2(dout)
  [board_id] -> pkt_reord0/7(board_id)
  pkt_reord0/8(xeng_id) -> [id0]
  pkt_reord1/1(sync_out) -> [x_sync1], Delay49/1(d), Subsystem1/1(sync), miss_cnt1/2(sync)
  pkt_reord1/2(data_out) -> Delay50/1(d), Subsystem1/2(dout)
  pkt_reord1/3(valid_out) -> miss_cnt1/3(valid), Delay51/1(d), Subsystem1/3(valid)
  pkt_reord1/4(mcnt_out) -> Delay52/1(d), Subsystem1/4(mcnt)
  pkt_reord0/6(flag_out) -> Subsystem14/5(flag)
  [board_id] -> pkt_reord1/7(board_id)
  pkt_reord1/8(xeng_id) -> [id1]
  pkt_reord1/5(rcvd) -> miss_cnt1/4(rcvd), Subsystem1/6(rcvd)
  [new_acc1] -> Logical2/1
  [new_acc0] -> Logical2/2
  Logical2/1 -> Delay118/1(d)
  Delay27/1(q) -> negedge_delay2/1
  Inverter/1 -> Delay27/1(d)
  [gbe_up] -> Inverter/1
  Delay96/1(q) -> negedge_delay3/1
  Logical4/1 -> Delay96/1(d)
  [xeng_err0] -> Logical4/1
  [xeng_err1] -> Logical4/2
  Delay97/1(q) -> negedge_delay4/1
  Logical5/1 -> Delay97/1(d)
  [vacc_err0] -> Logical5/1
  [vacc_err1] -> Logical5/2
  miss_cnt0/4(err_now) -> [xeng_err0]
  miss_cnt1/4(err_now) -> [xeng_err1]
  Constant10/1 -> Delay147/1(d)
  Constant11/1 -> Delay148/1(d)
  Constant16/1 -> miss_cnt0/5(err), [pkt_reord_err0]
  Constant17/1 -> miss_cnt1/5(err), [pkt_reord_err1]
  Subsystem1/1(data) -> Delay1/1(d)
  Subsystem1/2(we) -> Delay3/1(d)
  Subsystem1/3(trig) -> Delay4/1(d)
  Subsystem1/4(stop) -> Delay5/1(d)
  pkt_reord1/6(flag_out) -> Subsystem1/5(flag)
  vacc0/2(data_out) -> delay4/1
  vacc0/4(valid_out) -> delay2/1
  vacc0/6(rb_done) -> delay6/1
  vacc0/5(pkt_rdy) -> [vacc_pkt_rdy0]
  [vacc_ld_stat0] -> vacc_ld_status0/1
  vacc0/8(ld_status) -> [vacc_ld_stat0]
  [id0] -> pack_out0/6(xeng_id)
  [cnt_rst] -> vacc_monitor1/1(cnt_rst)
  [vacc_err1] -> vacc_monitor1/3(error)
  [new_acc1] -> vacc_monitor1/2(new_acc)
  vacc1/1(new_acc) -> [new_acc1]
  vacc1/2(data_out) -> delay16/1
  vacc1/4(valid_out) -> delay17/1
  vacc1/5(pkt_rdy) -> [vacc_pkt_rdy1]
  vacc1/6(rb_done) -> delay19/1
  vacc1/7(timestamp) -> delay20/1
  [id1] -> pack_out1/6(xeng_id)
  vacc1/8(ld_status) -> [vacc_ld_stat1]
  vacc1/10(error_out) -> [vacc_err1]
  [vacc_ld_stat1] -> vacc_ld_status1/1
  pack_out1/5(fetch) -> vacc1/9(re)
  [vacc_err0] -> snap_vacc0/4(stop)
  [gbe_out_enable] -> Logical1/2
  Logical1/1 -> [gbe_txvld]
  pack_out0/3(eof) -> Delay15/1(d)
  err_clear_sim1/1 -> gbe_out_port/1
  gbe_out_port/1 -> Delay9/1(d)
  Delay9/1(q) -> [gbe_out_txport]
  err_clear_sim5/1 -> gbe_out_ip/1
  gbe_out_ip/1 -> Delay10/1(d)
  Delay10/1(q) -> [gbe_out_ip_addr]
  Constant2/1 -> [gbe_rxack]
  [gbe_out_ip_addr] -> [gbe_txip]
  pack_out_err0/1 -> Terminator6/1
  pack_out_cnt0/1 -> Terminator5/1
  [cnt_rst] -> vacc_monitor2/1(cnt_rst)
  vacc_monitor2/1(pkt_cnt) -> pack_out_cnt0/1
  vacc_monitor2/2(error_cnt) -> pack_out_err0/1
  pack_out_err1/1 -> Terminator21/1
  pack_out_cnt1/1 -> Terminator12/1
  [cnt_rst] -> vacc_monitor3/1(cnt_rst)
  vacc_monitor3/1(pkt_cnt) -> pack_out_cnt1/1
  vacc_monitor3/2(error_cnt) -> pack_out_err1/1
  pack_out1/3(eof) -> Delay11/1(d)
  pack_out0/5(fetch) -> vacc0/9(re)
  delay4/1 -> snap_vacc0/1(din), pack_out0/1(data_in)
  delay2/1 -> snap_vacc0/2(we), pack_out0/2(valid_in)
  delay6/1 -> snap_vacc0/3(trig), pack_out0/4(rb_done)
  delay7/1 -> pack_out0/5(timestamp)
  delay16/1 -> pack_out1/1(data_in)
  delay17/1 -> pack_out1/2(valid_in)
  delay19/1 -> pack_out1/4(rb_done)
  delay20/1 -> pack_out1/5(timestamp)
  pack_out0/1(data_out) -> Delay23/1(d)
  pack_out0/2(valid_out) -> Delay31/1(d)
  Delay23/1(q) -> [vacc_dout0]
  Delay31/1(q) -> [vacc_valid0]
  Delay15/1(q) -> [vacc_eof0]
  Delay14/1(q) -> [vacc_dout1]
  Delay16/1(q) -> [vacc_valid1]
  Delay11/1(q) -> [vacc_eof1]
  [vacc_dout0] -> mux_out/1(din0)
  [vacc_valid0] -> mux_out/2(valid0)
  [vacc_eof0] -> mux_out/3(eof0)
  [vacc_pkt_rdy0] -> mux_out/4(data_rdy0)
  [vacc_dout1] -> mux_out/5(din1)
  [vacc_valid1] -> mux_out/6(valid1)
  [vacc_eof1] -> mux_out/7(eof1)
  [vacc_pkt_rdy1] -> mux_out/8(data_rdy1)
  mux_out/4(pkt_send0) -> [tx_pkt0]
  mux_out/5(pkt_send1) -> [tx_pkt1]
  pack_out1/1(data_out) -> Delay14/1(d)
  pack_out1/2(valid_out) -> Delay16/1(d)
  [vacc_eof1] -> vacc_monitor3/2(eof)
  Delay33/1(q) -> [pack_err1]
  pack_out1/4(err) -> Delay33/1(d)
  [pack_err1] -> vacc_monitor3/3(error)
  Delay34/1(q) -> [pack_err0]
  pack_out0/4(err) -> Delay34/1(d)
  [vacc_eof0] -> vacc_monitor2/2(eof)
  [pack_err0] -> vacc_monitor2/3(error)
  mux_out/1(dout) -> [gbe_txd]
  mux_out/2(valid) -> Logical1/1
  mux_out/3(eof) -> [gbe_txeof]
  delay8/1 -> gbe0/1
  delay9/1 -> gbe0/2
  delay10/1 -> gbe0/3
  delay11/1 -> gbe0/4
  delay12/1 -> gbe0/5
  delay13/1 -> gbe0/6
  delay14/1 -> gbe0/7
  delay15/1 -> gbe0/8
  [tx_pkt0] -> pack_out0/3(pkt_rdy)
  [tx_pkt1] -> pack_out1/3(pkt_rdy)
  Slice6/1 -> Delay44/1(d)
  Delay44/1(q) -> [flasher_en]
  Constant1/1 -> front_panel/5(led1_red)
  negedge_delay3/1 -> front_panel/6(led2_red)
  negedge_delay4/1 -> front_panel/7(led3_red)
  Constant15/1 -> front_panel/8(led4_green)
  Inverter1/1 -> led0_10gbe_fault/1
  Inverter2/1 -> led1_xaui_sync/1
  Inverter3/1 -> led2_x_sync/1
  Inverter4/1 -> led3_new_acc/1
  [flasher_en] -> front_panel/1(flasher_en)
  [new_acc0] -> front_panel/2(sync)
  [x_sync0] -> front_panel/3(step)
  xstatus0/1 -> Terminator24/1
  Subsystem6/1(status) -> xstatus0/1
  [clr_status] -> Subsystem6/1(clr)
  negedge_delay2/1 -> front_panel/4(led0_red), Inverter1/1
  negedge_delay/1 -> front_panel/10(led6_green), Inverter3/1
  negedge_delay1/1 -> front_panel/9(led5_green), Inverter4/1
  Constant5/1 -> front_panel/11(led7_green)
  Slice10/1 -> Delay13/1(d)
  Delay13/1(q) -> [clr_status]
  Inverter5/1 -> Subsystem6/2(gbe_down)
  [gbe_up] -> Inverter5/1
  [xeng_err0] -> Subsystem6/3(xeng_err)
  [vacc_err0] -> Subsystem6/4(vacc_err)
  [rx_bad_pkt] -> Subsystem6/5(rx_bad_pkt)
  [gbe_rx_bad] -> Subsystem6/6(rx_bad_frame)
  [gbe_tx_over] -> Subsystem6/7(tx_over)
  [pkt_reord_err0] -> Subsystem6/8(pkt_reord_err)
  [pack_err0] -> Subsystem6/9(pack_err)
  xstatus1/1 -> Terminator27/1
  Subsystem7/1(status) -> xstatus1/1
  [clr_status] -> Subsystem7/1(clr)
  Inverter6/1 -> Subsystem7/2(gbe_down)
  [gbe_up] -> Inverter6/1
  [xeng_err1] -> Subsystem7/3(xeng_err)
  [vacc_err1] -> Subsystem7/4(vacc_err)
  [rx_bad_pkt] -> Subsystem7/5(rx_bad_pkt)
  [gbe_rx_bad] -> Subsystem7/6(rx_bad_frame)
  [gbe_tx_over] -> Subsystem7/7(tx_over)
  [pkt_reord_err1] -> Subsystem7/8(pkt_reord_err)
  [pack_err1] -> Subsystem7/9(pack_err)
  Delay1/1(q) -> snap_descramble1/1(din)
  Delay3/1(q) -> snap_descramble1/2(we)
  Delay4/1(q) -> snap_descramble1/3(trig)
  Delay5/1(q) -> snap_descramble1/4(stop)
  Delay42/1(q) -> snap_descramble0/2(we)
  Delay43/1(q) -> snap_descramble0/3(trig)
  Subsystem3/1(data_out) -> snap_gbe_tx0/1(din)
  Subsystem3/2(we_out) -> snap_gbe_tx0/2(we)
  Subsystem3/3(trig_out) -> snap_gbe_tx0/3(trig)
  prep_snap_rx0/1(data_out) -> snap_rx0/1(din)
  prep_snap_rx0/2(we_out) -> snap_rx0/2(we)
  prep_snap_rx0/3(trig_out) -> snap_rx0/3(trig)
  Subsystem4/1(data_out) -> snap_gbe_rx0/1(din)
  Subsystem4/2(we_out) -> snap_gbe_rx0/2(we)
  Subsystem4/3(trig_out) -> snap_gbe_rx0/3(trig)
  Delay130/1(q) -> last_missing_ant0/1
  last_missing_ant0/1 -> Terminator11/1
  pkt_reord_cnt1/1 -> Terminator22/1
  pkt_reord_err1/1 -> Terminator23/1
  Delay32/1(q) -> pkt_reord_cnt1/1
  Delay45/1(q) -> pkt_reord_err1/1
  Delay28/1(q) -> last_missing_ant1/1
  last_missing_ant1/1 -> Terminator2/1
  miss_cnt1/1(spectr_cnt) -> Delay32/1(d)
  miss_cnt1/2(err_cnt) -> Delay45/1(d)
  miss_cnt1/3(last_missed_ant) -> Delay28/1(d)
